simulink model "Simulatore_COMAU_UBHAND_IV_manipolazione_interna"  (Simulink 7.6)
settings: Solver=ode45  StartTime=0.0  StopTime=inf  RelTol=1e-3
values from neighbouring files (symbols this model uses):
  a_f = zeros(4,5)    [KinematicData.m]
  alpha_f = zeros(4,5)    [KinematicData.m]
  d_f = zeros(4,5)    [KinematicData.m]
  dc4_1 = 660    [KinematicData.m]
  T6HAND = [1 0 0 0;0 1 0 0; 0 0 1 357.48+95; 0 0 0 1]    [KinematicData.m]
  teta_d = atan(647.07/130)    [KinematicData.m]
  teta_f = zeros(4,5)    [KinematicData.m]
undefined symbols (not defined in the file or in its folder): a_c, a_w, alpha_c, alpha_w, d_c, d_w, Dt_fingers, teta_c
libraries: simulink x281, dspmtrx3 x171, aerolibutil x163, aerolibconvert2 x64, simulink_extras x46, vrlib x26, aerolibtransform2 x7, dspnetwork x1
(part 1 of 15)
settings Model: Creator=UMBERTO  LastModifiedBy=root
settings OptimizationCC: InitFltsAndDblsToZero=on
settings DebuggingCC: StrictBusMsg=Warning

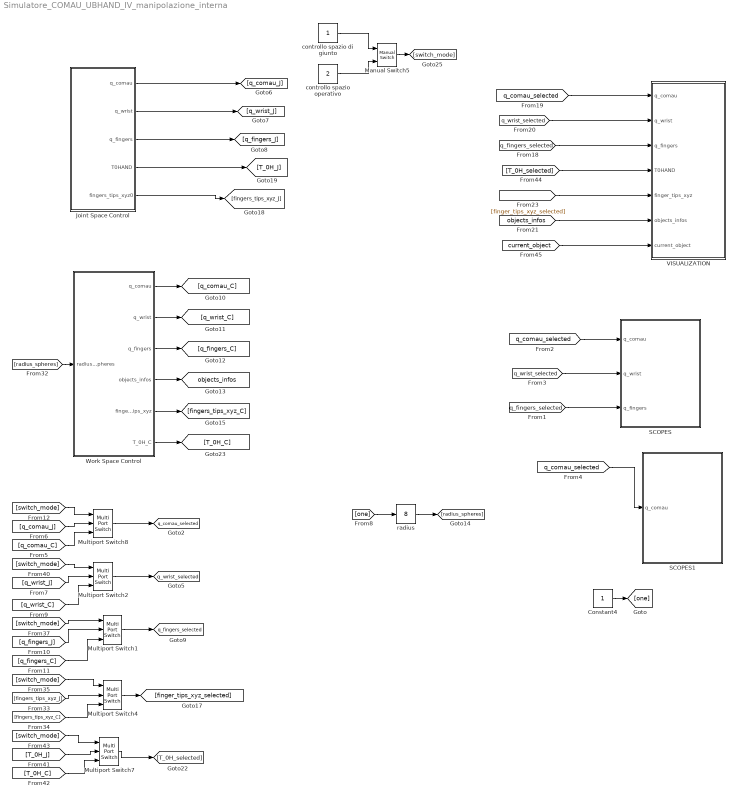
== system Simulatore_COMAU_UBHAND_IV_manipolazione_interna ==
  Constant4 : Constant
  Joint Space Control : Subsystem
  Manual Switch5 : simulink/Signal Routing/Manual Switch  SystemSampleTime=-1  sw=0  action=0  varsize=off
  Multiport Switch{1..2,4,7..8} (5 alike) : MultiPortSwitch  Inputs=2  zeroidx=off
  SCOPES : Subsystem
  SCOPES1 : Subsystem
  VISUALIZATION : Subsystem
  Work Space Control : Subsystem
  controllo spazio di giunto : Constant
  controllo spazio operativo : Constant  Value=2
  radius : simulink/Math Operations/Slider Gain  SystemSampleTime=-1  low=0  gain=8  high=200
  tag scope: objects_infos=global, q_comau_selected=global, q_fingers_selected=global, q_wrist_selected=global
  wires:
  Work Space Control/1(q_comau) -> [q_comau_C]
  Work Space Control/2(q_wrist) -> [q_wrist_C]
  Work Space Control/3(q_fingers) -> [q_fingers_C]
  Work Space Control/4(objects_infos) -> [objects_infos]
  [radius_spheres] -> Work Space Control/1(radius_spheres)
  Work Space Control/5(fingers_tips_xyz) -> [fingers_tips_xyz_C]
  Work Space Control/6(T_0H_C) -> [T_0H_C]
  Joint Space Control/1(q_comau) -> [q_comau_J]
  Joint Space Control/2(q_wrist) -> [q_wrist_J]
  Joint Space Control/3(q_fingers) -> [q_fingers_J]
  Joint Space Control/4(T0HAND) -> [T_0H_J]
  Joint Space Control/5(fingers_tips_xyz0) -> [fingers_tips_xyz_J]
  Multiport Switch8/1 -> [q_comau_selected]
  [q_comau_C] -> Multiport Switch8/3
  [q_wrist_J] -> Multiport Switch2/2
  [q_wrist_C] -> Multiport Switch2/3
  [q_fingers_J] -> Multiport Switch1/2
  [q_fingers_C] -> Multiport Switch1/3
  [switch_mode] -> Multiport Switch2/1
  [switch_mode] -> Multiport Switch1/1
  Multiport Switch1/1 -> [q_fingers_selected]
  Multiport Switch2/1 -> [q_wrist_selected]
  [fingers_tips_xyz_J] -> Multiport Switch4/2
  [fingers_tips_xyz_C] -> Multiport Switch4/3
  [switch_mode] -> Multiport Switch4/1
  Multiport Switch4/1 -> [finger_tips_xyz_selected]
  [T_0H_J] -> Multiport Switch7/2
  [T_0H_C] -> Multiport Switch7/3
  [switch_mode] -> Multiport Switch7/1
  Multiport Switch7/1 -> [T_0H_selected]
  [switch_mode] -> Multiport Switch8/1
  [q_comau_J] -> Multiport Switch8/2
  controllo spazio di giunto/1 -> Manual Switch5/1
  controllo spazio operativo/1 -> Manual Switch5/2
  Manual Switch5/1 -> [switch_mode]
  [q_comau_selected] -> VISUALIZATION/1(q_comau)
  [q_wrist_selected] -> VISUALIZATION/2(q_wrist)
  [q_fingers_selected] -> VISUALIZATION/3(q_fingers)
  [T_0H_selected] -> VISUALIZATION/4(T0HAND)
  [finger_tips_xyz_selected] -> VISUALIZATION/5(finger_tips_xyz)
  [one] -> radius/1
  radius/1 -> [radius_spheres]
  Constant4/1 -> [one]
  [q_comau_selected] -> SCOPES/1(q_comau)
  [q_wrist_selected] -> SCOPES/2(q_wrist)
  [q_fingers_selected] -> SCOPES/3(q_fingers)
  [objects_infos] -> VISUALIZATION/6(objects_infos)
  [current_object] -> VISUALIZATION/7(current_object)
  [q_comau_selected] -> SCOPES1/1(q_comau)

== system Simulatore_COMAU_UBHAND_IV_manipolazione_interna/Joint Space Control ==
  Constant : Constant
  Direct_kinematic2 : Subsystem
  Display : Display
  Mux : Mux  Inputs=6
  Mux1 : Mux  Inputs=5
  Sinusoidal Signals_fingers_wrist : Subsystem
  Step Signals fingers_wrist : Subsystem
  Switch finger references : simulink/Signal Routing/Manual Switch  sw=1  action=0  varsize=off
  Switch wrist : simulink/Signal Routing/Manual Switch  sw=1  action=0  varsize=off
  Switch{2..7} (6 alike) : simulink/Signal Routing/Manual Switch  sw=1  action=0  varsize=off
  q1 : simulink/Math Operations/Slider Gain  low=-170  gain=0  high=170
  q1_sin : Sin  Amplitude=20  Frequency=0.1  SampleTime=0
  q2 : simulink/Math Operations/Slider Gain  low=-85  gain=0  high=155
  q2_sin : Sin  Amplitude=50  Bias=20  SampleTime=0
  q3 : simulink/Math Operations/Slider Gain  low=-170  gain=-90  high=0
  q3_sin : Sin  Amplitude=35  Bias=45  Frequency=0.3  SampleTime=0
  q4 : simulink/Math Operations/Slider Gain  low=-210  gain=0  high=210
  q4_sin : Sin  Amplitude=90  Frequency=0.3  SampleTime=0
  q5 : simulink/Math Operations/Slider Gain  low=-130  gain=90  high=920
  q5_sin : Sin  Amplitude=45  Bias=45  Frequency=0.3  SampleTime=0
  q6 : simulink/Math Operations/Slider Gain  low=-2700  gain=0  high=2700
  q6_sin : Sin  Amplitude=45  Bias=45  Frequency=5  SampleTime=0
  q_comau : Outport
  q_wrist : Outport  Port=2
  q_fingers : Outport  Port=3
  T0HAND : Outport  Port=4
  fingers_tips_xyz0 : Outport  Port=5  PortDimensions=20
  wires:
  q6_sin/1 -> Switch7/2
  q5_sin/1 -> Switch6/2
  q4_sin/1 -> Switch5/2
  q3_sin/1 -> Switch4/2
  q2_sin/1 -> Switch3/2
  q1_sin/1 -> Switch2/2
  Mux/1 -> [q]
  Switch2/1 -> Mux/1
  Switch3/1 -> Mux/2
  Switch4/1 -> Mux/3
  Switch5/1 -> Mux/4
  Switch6/1 -> Mux/5
  Switch7/1 -> Mux/6
  Sinusoidal Signals_fingers_wrist/1(wrist) -> Switch wrist/2
  Switch wrist/1 -> [wrist]
  Sinusoidal Signals_fingers_wrist/2(fingers_angles) -> Switch finger references/2
  [one] -> q1/1
  Constant/1 -> [one]
  q1/1 -> Switch2/1
  [one] -> q2/1
  q2/1 -> Switch3/1
  [one] -> q3/1
  q3/1 -> Switch4/1
  [one] -> q4/1
  [one] -> q5/1
  [one] -> q6/1
  q4/1 -> Switch5/1
  q5/1 -> Switch6/1
  q6/1 -> Switch7/1
  Step Signals fingers_wrist/1(fingers_angles) -> Switch finger references/1
  Step Signals fingers_wrist/2(wrist) -> Switch wrist/1
  [q] -> q_comau/1
  [wrist] -> q_wrist/1
  [fingers] -> q_fingers/1
  Switch finger references/1 -> [fingers], Display/1
  [q] -> Direct_kinematic2/1(q[1..6])
  Direct_kinematic2/1(T0HAND) -> T0HAND/1
  [wrist] -> Direct_kinematic2/2(wrist_angles[1..2])
  [fingers] -> Direct_kinematic2/3(fingers_joints_references)
  Direct_kinematic2/2(T_finger_tip_thumb) -> Mux1/1
  Direct_kinematic2/6(T_finger_tip_little) -> Mux1/5
  Direct_kinematic2/5(T_finger_tip_ring) -> Mux1/4
  Direct_kinematic2/4(T_finger_tip_middle) -> Mux1/3
  Direct_kinematic2/3(T_finger_tip_index) -> Mux1/2
  Mux1/1 -> fingers_tips_xyz0/1

== system Simulatore_COMAU_UBHAND_IV_manipolazione_interna/SCOPES ==
  q_comau : Inport
  q_wrist : Inport  Port=2
  q_fingers : Inport  Port=3
  Demux : Demux  Outputs=5
  Demux1 : Demux  Outputs=5
  Demux2 : Demux  Outputs=6
  Direct_kinematic2 : Subsystem
  Scope : Scope  NumInputPorts=6
      settings: YMin=-70~-50~-150~-110~-90~125  YMax=-10~30~-100~-50~-20~160
  T_0HAND : Display
  T_finger_tip_index : Display
  T_finger_tip_little : Display
  T_finger_tip_middle : Display
  T_finger_tip_ring : Display
  T_finger_tip_thumb : Display
  q[1..2] wrist : Display
  q[1..4] index : Display
  q[1..4] little : Display
  q[1..4] middle : Display
  q[1..4] ring : Display
  q[1..4] thumb : Display
  q[1..6] : Display
  tag scope: T_0H=global
  wires:
  Demux/1 -> T_finger_tip_thumb/1
  Demux/2 -> T_finger_tip_index/1
  Demux/3 -> T_finger_tip_middle/1
  Demux/4 -> T_finger_tip_ring/1
  Demux/5 -> T_finger_tip_little/1
  q_comau/1 -> q[1..6]/1, Direct_kinematic2/1(q[1..6]), Demux2/1
  q_wrist/1 -> q[1..2] wrist/1, Direct_kinematic2/2(wrist_angles[1..2])
  Direct_kinematic2/2(fingers_tips_xyz) -> Demux/1
  q_fingers/1 -> Demux1/1, Direct_kinematic2/3(fingers_joints_references)
  Demux1/1 -> q[1..4] thumb/1
  Demux1/2 -> q[1..4] index/1
  Demux1/3 -> q[1..4] middle/1
  Demux1/4 -> q[1..4] ring/1
  Demux1/5 -> q[1..4] little/1
  Direct_kinematic2/1(T0HAND) -> T_0HAND/1, [T_0H]
  Demux2/1 -> Scope/1
  Demux2/2 -> Scope/2
  Demux2/3 -> Scope/3
  Demux2/6 -> Scope/6
  Demux2/4 -> Scope/4
  Demux2/5 -> Scope/5

== system Simulatore_COMAU_UBHAND_IV_manipolazione_interna/SCOPES1 ==
  q_comau : Inport
  Demux2 : Demux  Outputs=6
  Detect Change : simulink/Logic and Bit Operations/Detect Change  vinit=[6 0 0 0 0 0 0]  InputProcessing=Inherited  OutDataTypeStr=uint8
  KILL1 : Constant  Value=[6 0 0 0 0 0 0]
  Logical Operator : Logic  AllPortsSameDT=off  OutDataTypeStr=boolean
  Manual Switch1 : simulink/Signal Routing/Manual Switch  sw=0  action=0  varsize=off
  Manual Switch5 : simulink/Signal Routing/Manual Switch  sw=1  action=0  varsize=off
  Mux : Mux  Inputs=2
  NOT SEND : Constant  Value=0
  SEND : Constant
  SEND CONFIGURATION : Constant  Value=6
  Scope : Scope  NumInputPorts=6
      settings: YMin=-80~0~-175~-90~-78~80  YMax=20~100~0~20~-71~170
  Subsystem : Subsystem
  q[1..6] : Display
  wires:
  q_comau/1 -> q[1..6]/1, Demux2/1, Mux/2
  Demux2/1 -> Scope/1
  Demux2/2 -> Scope/2
  Demux2/3 -> Scope/3
  Demux2/6 -> Scope/6
  Demux2/4 -> Scope/4
  Demux2/5 -> Scope/5
  NOT SEND/1 -> Manual Switch5/1
  SEND/1 -> Manual Switch5/2
  Manual Switch5/1 -> Logical Operator/1
  Manual Switch1/1 -> Detect Change/1, Subsystem/1(DATA_TO_COMAU)
  SEND CONFIGURATION/1 -> Mux/1
  KILL1/1 -> Manual Switch1/1
  Mux/1 -> Manual Switch1/2
  Detect Change/1 -> Logical Operator/2
  Logical Operator/1 -> Subsystem/Enable

== system Simulatore_COMAU_UBHAND_IV_manipolazione_interna/VISUALIZATION ==
  q_comau : Inport
  q_wrist : Inport  Port=2
  q_fingers : Inport  Port=3
  T0HAND : Inport  Port=4
  finger_tips_xyz : Inport  Port=5
  objects_infos : Inport  Port=6
  current_object : Inport  Port=7
  Manual Switch : simulink/Signal Routing/Manual Switch  SystemSampleTime=-1  sw=0  action=0  varsize=off
  Multiport Switch1 : MultiPortSwitch  Inputs=2  zeroidx=off
  Subsystem : Subsystem
  Subsystem1 : Subsystem
  Subsystem2 : Subsystem
  Subsystem3 : Subsystem
  Subsystem4 : Subsystem
  Subsystem5 : Subsystem
  Subsystem6 : Subsystem
  Subsystem9 : Subsystem
  VR Sink1 : vrlib/VR Sink  InstantiateOnLoad=on  SampleTime=0.1  ViewEnable=on  RemoteChange=off  RemoteView=off  FieldsWritten=primo_giunto.rotation.4.1.1.double#secondo_giunto.rotation.4.1.1.double#terzo_giunto.rotation.4.1.1.double#quarto_giunto.rotation.4.1.1.double#quinto_giunto.rotation.4.1.1.double#sesto_giunto.rotation.4.1.1.double#PRIMO_GIUNTO_POLSO.rotation.4.1.1.double#SECONDO_GIUNTO_POLSO.rotation.4.1.1.double#CARPALE_POLLICE.rotation.4.1.1.double#PROSSIMALE_POLLICE.rotation.4.1.1.double#MEDIALE_POLLICE.rotation.4.1.1.double#DISTALE_POLLICE.rotation.4.1.1.double#CARPALE_INDICE.rotation.4.1.1.double#PROSSIMALE_INDICE.rotation.4.1.1.double#MEDIALE_INDICE.rotation.4.1.1.double#DISTALE_INDICE.rotation.4.1.1.double#CARPALE_MEDIO.rotation.4.1.1.double#PROSSIMALE_MEDIO.rotation.4.1.1.double#MEDIALE_MEDIO.rotation.4.1.1.double#DISTALE_MEDIO.rotation.4.1.1.double#CARPALE_ANULARE.rotation.4.1.1.double#PROSSIMALE_ANULARE.rotation.4.1.1.double#MEDIALE_ANULARE.rotation.4.1.1.double#DISTALE_ANULARE.rotation.4.1.1.double#CARPALE_MIGNOLO.rotation.4.1.1.double#PROSSIMALE_MIGNOLO.rotation.4.1.1.double#MEDIALE_MIGNOLO.rotation.4.1.1.double#DISTALE_MIGNOLO.rotation.4.1.1.double#comau_wrist.rotation.4.1.1.double#comau_wrist.translation.3.1.1.double#mignolo_punta.translation.3.1.1.double#anulare_punta.translation.3.1.1.double#medio_punta.translation.3.1.1.double#pollice_punta.translation.3.1.1.double#indice_punta.translation.3.1.1.double#system_reference_index.rotation.4.1.1.double#system_reference_index.translation.3.1.1.double#sfera.translation.3.1.1.double#sfera_size.radius.1.1.1.double#parallelepipedo.rotation.4.1.1.double#parallelepipedo.translation.3.1.1.double#parallelepipedo_size.size.3.1.1.double#cilindro.rotation.4.1.1.double#cilindro.translation.3.1.1.double#cilindro_size.height.1.1.1.double#cilindro_size.radius.1.1.1.double#mignolo_contact_point.translation.3.1.1.double#anulare_contact_point.translation.3.1.1.double#medio_contact_point.translation.3.1.1.double#pollice_contact_point.translation.3.1.1.double#indice_contact_point.translation.3.1.1.double#system_reference_1.rotation.4.1.1.double#system_reference_1.translation.3.1.1.double#system_reference_2.rotation.4.1.1.double#system_reference_2.translation.3.1.1.double#system_reference_3.rotation.4.1.1.double#system_reference_3.translation.3.1.1.double#system_reference_4.rotation.4.1.1.double#system_reference_4.translation.3.1.1.double  WorldFileName=COMAU_UBHAND_IV_cascata.WRL  WorldDescription=C:\Documents and Settings\Umberto\Documenti\Tesi\smart_six_vrml\vrml\SMART SiX_base.wrl  AutoView=on  VideoDimensions=[]  FigureProperties="{'Position', 'Name', 'Viewpoint', 'CameraBound', 'CameraDirection', 'CameraPosition', 'CameraUpVector', 'ZoomFactor', 'Antialiasing', 'Headlight', 'Lighting', 'NavPanel', 'StatusBar', 'ToolBar', 'Textures', 'Transparency', 'Wireframe', 'NavZones', 'NavMode', 'NavSpeed', 'Record2DCompressMethod', 'Record2DCompressQuality', 'Record2D', 'Record2DFileName', 'Record2DFPS'; [1.6600000000000000e+02   1.5200000000000000e+02   1.1560000000000000e+03   5.4000000000000000e+02], 'C:\\Documents and Settings\\Umberto\\Documenti\\Tesi\\smart_six_vrml\\vrml\\SMART SiX_base.wrl', 'vista_generale_da avanti', 'on', [1.3025964760090943e-02  -9.4132527063624802e-04  -9.9991471543767951e-01], [5.1898779160484864e+01   8.1516929012152815e+01  -2.5729076904413084e+01], [5.0428377425442407e-02   9.9872763980294033e-01  -2.8327345742631266e-04], [6.2092132305915493e-01], 'on', 'on', 'on', 'halfbar', 'on', 'on', 'on', 'on', 'off', 'off', 'fly', 'normal', 'auto', [7.5000000000000000e+01], 'off', '%f_anim_%n.avi', [1.5000000000000000e+01]}"
  current_object1 : InportShadow  Port=7
  wires:
  q_wrist/1 -> Subsystem3/2(q_wrist)
  q_fingers/1 -> Subsystem3/3(q_fingers)
  q_comau/1 -> Subsystem3/1(q_comau)
  T0HAND/1 -> Subsystem3/4(T_0H)
  objects_infos/1 -> Subsystem1/1(Object Infos)
  finger_tips_xyz/1 -> Subsystem3/5(finger_tips_xyz)
  current_object/1 -> Subsystem1/2(Current_object)
  Multiport Switch1/1 -> Subsystem9/1(xyz_fingers)
  [fingers_tips_xyz] -> Subsystem2/1(finger_tips_reference_into_finger_frame)
  [reference_mode] -> Multiport Switch1/1
  [T_0_index] -> Subsystem/1(T_0_index)
  current_object1/1 -> Subsystem4/1(Current_object)
  [T_0H] -> Subsystem5/1(T_0HAND)
  Subsystem2/1(finger_tips_into_base_frame) -> Manual Switch/1
  Manual Switch/1 -> Multiport Switch1/2
  Subsystem3/1(q1_comau) -> VR Sink1/1
  Subsystem3/2(q2_comau) -> VR Sink1/2
  Subsystem3/3(q3_comau) -> VR Sink1/3
  Subsystem3/4(q4_comau) -> VR Sink1/4
  Subsystem3/5(q_5_comau) -> VR Sink1/5
  Subsystem3/6(q_6_comau) -> VR Sink1/6
  Subsystem3/7(polso_1) -> VR Sink1/7
  Subsystem3/8(polso_2) -> VR Sink1/8
  Subsystem3/9(carpale_pollice) -> VR Sink1/9
  Subsystem3/10(prossimale_pollice) -> VR Sink1/10
  Subsystem3/11(mediale_pollice) -> VR Sink1/11
  Subsystem3/12(distale_pollice) -> VR Sink1/12
  Subsystem3/13(carplae_indice) -> VR Sink1/13
  Subsystem3/14(prossimale_indice) -> VR Sink1/14
  Subsystem3/15(mediale_indice) -> VR Sink1/15
  Subsystem3/16(distale_indice) -> VR Sink1/16
  Subsystem3/17(carpale_medio) -> VR Sink1/17
  Subsystem3/18(prossimale_medio) -> VR Sink1/18
  Subsystem3/19(mediale_medio) -> VR Sink1/19
  Subsystem3/20(distale_medio) -> VR Sink1/20
  Subsystem3/21(carpale_anulare) -> VR Sink1/21
  Subsystem3/22(prossimale_anulare) -> VR Sink1/22
  Subsystem3/23(mediale_anulare) -> VR Sink1/23
  Subsystem3/24(distale_anulare) -> VR Sink1/24
  Subsystem3/25(carpale_mignolo) -> VR Sink1/25
  Subsystem3/26(prossimale_mignolo) -> VR Sink1/26
  Subsystem3/27(mediale_mignolo) -> VR Sink1/27
  Subsystem3/28(distale_mignolo) -> VR Sink1/28
  Subsystem3/29(T_0H_ax/angle) -> VR Sink1/29
  Subsystem3/30(T_0H_xyz) -> VR Sink1/30
  Subsystem3/31(little_xyz) -> VR Sink1/31
  Subsystem3/32(ring_xyz) -> VR Sink1/32
  Subsystem3/33(middle_xyz) -> VR Sink1/33
  Subsystem3/34(thumb_xyz) -> VR Sink1/34
  Subsystem3/35(index_xyz) -> VR Sink1/35
  Subsystem1/1(Sphere_xyz) -> VR Sink1/38
  Subsystem1/2(sphere_radius) -> VR Sink1/39
  Subsystem1/3(Box_rotation) -> VR Sink1/40
  Subsystem1/4(Box_xyz) -> VR Sink1/41
  Subsystem1/5(Box_size) -> VR Sink1/42
  Subsystem1/6(Cylinder_rotation) -> VR Sink1/43
  Subsystem1/7(Cyliner_xyz) -> VR Sink1/44
  Subsystem1/8(Cylinder height) -> VR Sink1/45
  Subsystem1/9(Cylinder radius) -> VR Sink1/46
  Subsystem9/1(xyz_vrl_little) -> VR Sink1/47
  Subsystem9/2(xyz_vrl_ring) -> VR Sink1/48
  Subsystem9/3(xyz_vrl_middle) -> VR Sink1/49
  Subsystem9/4(xyz_vrl_thumb) -> VR Sink1/50
  Subsystem9/5(xyz_vrl_index) -> VR Sink1/51
  Subsystem4/4(T_0_rotation_frame_xyz) -> VR Sink1/57
  Subsystem4/3(T_0_rotation_frame_ax/angle) -> VR Sink1/56
  [fingers_tips_xyz_manip] -> Multiport Switch1/3
  Subsystem4/5(T_0_reference_object_frame_ax/angle) -> VR Sink1/58
  Subsystem4/6(T_0_reference_object_frame_xyz) -> VR Sink1/59
  Subsystem4/2(T_0EE_xyz) -> VR Sink1/55
  Subsystem4/1(T_0EE_ax_angle) -> VR Sink1/54
  [T_rot_center_cilindro_manip] -> Subsystem6/1(T_0HAND)
  Subsystem6/1(T_0HAND_ax/angle) -> VR Sink1/52
  Subsystem6/2(T_0HAND_xyz) -> VR Sink1/53

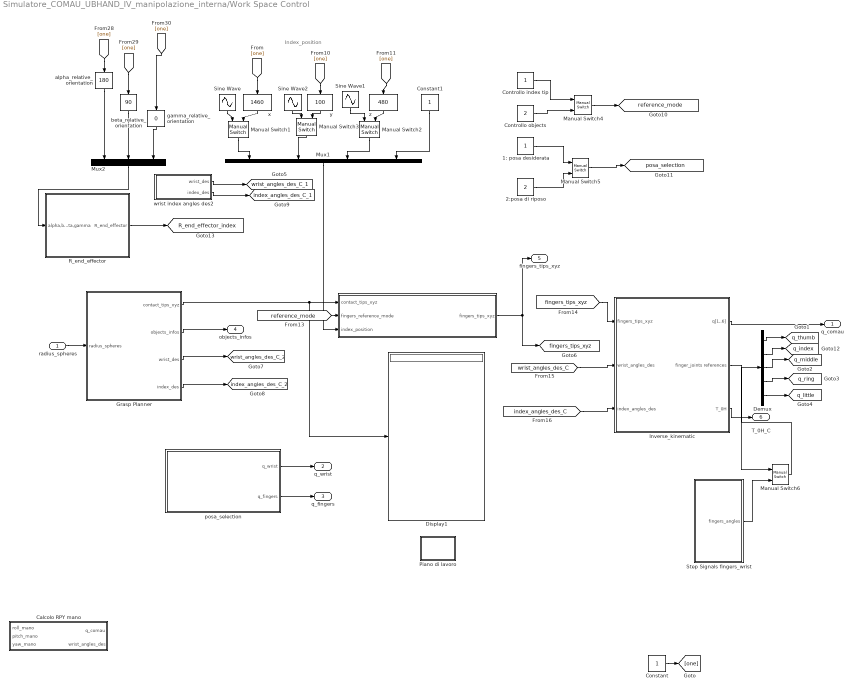
== system Simulatore_COMAU_UBHAND_IV_manipolazione_interna/Work Space Control ==
  note: Index_position
  radius_spheres : Inport
  " 2:posa di riposo" : Constant  Value=2
  " " : Subsystem
  1: posa desiderata : Constant
  Calcolo RPY mano : Subsystem
  Constant : Constant
  Constant1 : Constant
  Controllo index tip : Constant
  Controllo objects : Constant  Value=2
  Demux : Demux  Outputs=5
  Display1 : Display
  Grasp Planner : Subsystem
  Inverse_kinematic : Subsystem
  Manual Switch{1..4} (4 alike) : simulink/Signal Routing/Manual Switch  sw=0  action=0  varsize=off
  Manual Switch5 : simulink/Signal Routing/Manual Switch  sw=1  action=0  varsize=off
  Manual Switch6 : simulink/Signal Routing/Manual Switch  sw=1  action=0  varsize=off
  Mux1 : Mux
  Mux2 : Mux  Inputs=3
  Piano di lavoro : Subsystem
  R_end_effector : Subsystem
  Sine Wave : Sin  Amplitude=40  Bias=452.12  SampleTime=0
  Sine Wave1 : Sin  Amplitude=40  Bias=1200  Phase=pi/2  SampleTime=0
  Sine Wave2 : Sin  Amplitude=100  SampleTime=0
  Step Signals fingers_wrist : Subsystem
  alpha_relative_orientation : simulink/Math Operations/Slider Gain  low=-180  gain=180  high=180
  beta_relative_orientation : simulink/Math Operations/Slider Gain  low=-180  gain=90  high=180
  gamma_relative_orientation : simulink/Math Operations/Slider Gain  low=-180  gain=0  high=180
  posa_selection : Subsystem
  wrist index angles des2 : Subsystem
  x : simulink/Math Operations/Slider Gain  low=-200  gain=1460  high=2000
  y : simulink/Math Operations/Slider Gain  low=-2000  gain=100  high=2000
  z : simulink/Math Operations/Slider Gain  low=-1000  gain=480  high=2000
  q_comau : Outport
  q_wrist : Outport  Port=2
  q_fingers : Outport  Port=3
  objects_infos : Outport  Port=4
  fingers_tips_xyz : Outport  Port=5
  T_0H_C : Outport  Port=6
  tag scope: R_end_effector_index=global, fingers_tips_xyz=global, index_angles_des_C_1=global, index_angles_des_C_2=global, posa_selection=global, q_index=global, q_little=global, q_middle=global, q_ring=global, q_thumb=global, reference_mode=global, wrist_angles_des_C_1=global, wrist_angles_des_C_2=global
  wires:
  Constant/1 -> [one]
  Inverse_kinematic/1(q[1..6]) -> q_comau/1
  Grasp Planner/2(objects_infos) -> objects_infos/1
  Grasp Planner/1(contact_tips_xyz) -> " "/1(contact_tips_xyz), Display1/1
  " "/1(fingers_tips_xyz) -> fingers_tips_xyz/1, [fingers_tips_xyz]
  Demux/1 -> [q_thumb]
  radius_spheres/1 -> Grasp Planner/1(radius_spheres)
  Inverse_kinematic/3(T_0H) -> T_0H_C/1
  Grasp Planner/3(wrist_des) -> [wrist_angles_des_C_2]
  Grasp Planner/4(index_des) -> [index_angles_des_C_2]
  [one] -> z/1
  [one] -> y/1
  [one] -> x/1
  Sine Wave/1 -> Manual Switch1/1
  x/1 -> Manual Switch1/2
  Manual Switch1/1 -> Mux1/1
  Sine Wave1/1 -> Manual Switch2/1
  z/1 -> Manual Switch2/2
  Manual Switch2/1 -> Mux1/3
  Sine Wave2/1 -> Manual Switch3/1
  Manual Switch3/1 -> Mux1/2
  y/1 -> Manual Switch3/2
  Constant1/1 -> Mux1/4
  Mux1/1 -> " "/3(index_position)
  [reference_mode] -> " "/2(fingers_reference_mode)
  [fingers_tips_xyz] -> Inverse_kinematic/1("fingers_tips_xyz ")
  [wrist_angles_des_C] -> Inverse_kinematic/2(wrist_angles_des)
  [index_angles_des_C] -> Inverse_kinematic/3(index_angles_des)
  Controllo objects/1 -> Manual Switch4/2
  Controllo index tip/1 -> Manual Switch4/1
  Manual Switch4/1 -> [reference_mode]
  wrist index angles des2/1(wrist_des) -> [wrist_angles_des_C_1]
  wrist index angles des2/2(index_des) -> [index_angles_des_C_1]
  gamma_relative_orientation/1 -> Mux2/3
  beta_relative_orientation/1 -> Mux2/2
  alpha_relative_orientation/1 -> Mux2/1
  [one] -> gamma_relative_orientation/1
  [one] -> beta_relative_orientation/1
  [one] -> alpha_relative_orientation/1
  Mux2/1 -> R_end_effector/1(alpha,beta,gamma)
  R_end_effector/1(R_end_effector) -> [R_end_effector_index]
  Manual Switch5/1 -> [posa_selection]
  1: posa desiderata/1 -> Manual Switch5/1
  " 2:posa di riposo"/1 -> Manual Switch5/2
  posa_selection/1(q_wrist) -> q_wrist/1
  posa_selection/2(q_fingers) -> q_fingers/1
  Inverse_kinematic/2(finger_joints references) -> Manual Switch6/1
  Step Signals fingers_wrist/1(fingers_angles) -> Manual Switch6/2
  Manual Switch6/1 -> Demux/1
  Demux/2 -> [q_index]
  Demux/3 -> [q_middle]
  Demux/4 -> [q_ring]
  Demux/5 -> [q_little]

== system Simulatore_COMAU_UBHAND_IV_manipolazione_interna/Joint Space Control/Direct_kinematic2 ==
  q[1..6] : Inport
  wrist_angles[1..2] : Inport  Port=2
  fingers_joints_references : Inport  Port=3
  Constant2 : Constant  Value=T6HAND  VectorParams1D=off
  Direct_kinematic_Comau : Subsystem
  Direct_kinematic_hand : Subsystem
  Direct_kinematic_wrist : Subsystem
  Matrix Multiply1 : Product  Multiplication=Matrix(*)
  T0HAND : Outport
  T_finger_tip_thumb : Outport  Port=2
  T_finger_tip_index : Outport  Port=3
  T_finger_tip_middle : Outport  Port=4
  T_finger_tip_ring : Outport  Port=5
  T_finger_tip_little : Outport  Port=6
  wires:
  q[1..6]/1 -> Direct_kinematic_Comau/1(q[1..6])
  Direct_kinematic_Comau/1(T_0_6) -> Matrix Multiply1/1
  Constant2/1 -> Matrix Multiply1/2
  Matrix Multiply1/1 -> Direct_kinematic_wrist/2(T_0HAND), T0HAND/1
  wrist_angles[1..2]/1 -> Direct_kinematic_wrist/1(wrist_angles[1..2])
  fingers_joints_references/1 -> Direct_kinematic_hand/1(Joint_references)
  Direct_kinematic_hand/1(T_finger_tip_thumb) -> T_finger_tip_thumb/1
  Direct_kinematic_hand/2(T_finger_tip_index) -> T_finger_tip_index/1
  Direct_kinematic_hand/3(T_finger_tip_middle) -> T_finger_tip_middle/1
  Direct_kinematic_hand/4(T_finger_tip_ring) -> T_finger_tip_ring/1
  Direct_kinematic_hand/5(T_finger_tip_little) -> T_finger_tip_little/1
  Direct_kinematic_wrist/1(T_08) -> Direct_kinematic_hand/2(T_08)

== system Simulatore_COMAU_UBHAND_IV_manipolazione_interna/Joint Space Control/Sinusoidal Signals_fingers_wrist ==
  Index_medial : Sin  Amplitude=45  Bias=45  Phase=3/2*pi  SampleTime=0
  Index_proximal_back_forward : Sin  Amplitude=45  Bias=45  Phase=3/2*pi  SampleTime=0
  Index_proximal_left_right : Constant  Value=0
  Little_medial : Sin  Amplitude=0  Phase=3/2*pi  SampleTime=0
  Little_proximal_back_forward : Sin  Amplitude=0  Phase=3/2*pi  SampleTime=0
  Little_proximal_left_right : Constant  Value=0
  Middle_medial : Sin  Amplitude=0  Phase=3/2*pi  SampleTime=0
  Middle_proximal_back_forward : Sin  Amplitude=0  Phase=3/2*pi  SampleTime=0
  Middle_proximal_left_right : Constant  Value=0
  Mux{1..5,7} (6 alike) : Mux  Inputs=3
  Mux6 : Mux  Inputs=2
  Mux8 : Mux  Inputs=5
  Mux9 : Mux  Inputs=5
  Ring_medial : Sin  Amplitude=0  Phase=3/2*pi  SampleTime=0
  Ring_proximal_back_forward : Sin  Amplitude=0  Phase=3/2*pi  SampleTime=0
  Ring_proximal_left_right : Constant  Value=0
  Switch wrist : simulink/Signal Routing/Manual Switch  sw=0  action=0  varsize=off
  Thumb_medial : Sin  Amplitude=0  Phase=3/2*pi  SampleTime=0
  Thumb_medial1 : Sin  Amplitude=0  Phase=3/2*pi  SampleTime=0
  Thumb_proximal_back_forward : Sin  Amplitude=35  Bias=35  Phase=3/2*pi  SampleTime=0
  Thumb_proximal_back_forward1 : Sin  Amplitude=45  Bias=45  Phase=3/2*pi  SampleTime=0
  Thumb_proximal_left_right : Constant  Value=0
  Thumb_proximal_left_right1 : Sin  Amplitude=20  Phase=3/2*pi  SampleTime=0
  Wrist_back_forward : Sin  Amplitude=30  SampleTime=0
  Wrist_left_right : Sin  Amplitude=30  Phase=pi/2  SampleTime=0
  wrist : Outport
  fingers_angles : Outport  Port=2
  wires:
  Thumb_proximal_left_right/1 -> Mux1/1
  Thumb_medial/1 -> Mux1/3
  Mux6/1 -> wrist/1
  Mux1/1 -> [thumb], Mux9/1
  Thumb_proximal_back_forward/1 -> Mux1/2
  Index_proximal_left_right/1 -> Mux2/1
  Index_medial/1 -> Mux2/3
  Mux2/1 -> [index]
  Index_proximal_back_forward/1 -> Mux2/2
  Middle_proximal_left_right/1 -> Mux3/1
  Middle_medial/1 -> Mux3/3
  Mux3/1 -> [middle]
  Middle_proximal_back_forward/1 -> Mux3/2
  Ring_proximal_left_right/1 -> Mux4/1
  Ring_medial/1 -> Mux4/3
  Mux4/1 -> [ring]
  Ring_proximal_back_forward/1 -> Mux4/2
  Little_proximal_left_right/1 -> Mux5/1
  Little_medial/1 -> Mux5/3
  Mux5/1 -> [little]
  Little_proximal_back_forward/1 -> Mux5/2
  Wrist_left_right/1 -> Mux6/1
  Wrist_back_forward/1 -> Mux6/2
  Mux7/1 -> Mux9/3, Mux9/2, Mux9/4, Mux9/5
  Thumb_medial1/1 -> Mux7/3
  Thumb_proximal_back_forward1/1 -> Mux7/2
  Mux9/1 -> Switch wrist/2
  Mux8/1 -> Switch wrist/1
  Switch wrist/1 -> fingers_angles/1
  [thumb] -> Mux8/1
  [index] -> Mux8/2
  [middle] -> Mux8/3
  [ring] -> Mux8/4
  [little] -> Mux8/5
  Thumb_proximal_left_right1/1 -> Mux7/1

== system Simulatore_COMAU_UBHAND_IV_manipolazione_interna/Joint Space Control/Step Signals fingers_wrist ==
  All_fingers_together_back_forward : Constant  Value=0
  All_fingers_together_medial : Constant  Value=0
  All_fingers_together_proximal_left_right : Constant  Value=0
  Index_medial : Constant  Value=40
  Index_proximal_back_forward : Constant  Value=30
  Index_proximal_left_right : Constant  Value=5
  Little_medial : Constant  Value=0
  Little_proximal_back_forward : Constant  Value=0
  Little_proximal_left_right : Constant  Value=0
  Middle_medial : Constant  Value=30
  Middle_proximal_back_forward : Constant  Value=45
  Middle_proximal_left_right : Constant  Value=0
  Mux{1..5,7} (6 alike) : Mux  Inputs=3
  Mux6 : Mux  Inputs=2
  Mux8 : Mux  Inputs=5
  Mux9 : Mux  Inputs=5
  Ring_medial : Constant  Value=0
  Ring_proximal_back_forward : Constant  Value=0
  Ring_proximal_left_right : Constant  Value=0
  Switch wrist : simulink/Signal Routing/Manual Switch  sw=0  action=0  varsize=off
  Thumb_medial : Constant  Value=0
  Thumb_proximal_back_forward : Constant  Value=50
  Thumb_proximal_left_right : Constant  Value=0
  Wrist_back_forward1 : Constant  Value=0
  Wrist_left_right : Constant  Value=0
  fingers_angles : Outport
  wrist : Outport  Port=2
  tag scope: index_angles_des_J=global
  wires:
  Mux6/1 -> wrist/1
  Index_proximal_left_right/1 -> Mux2/1
  Index_medial/1 -> Mux2/3
  Index_proximal_back_forward/1 -> Mux2/2
  Middle_proximal_left_right/1 -> Mux3/1
  Middle_medial/1 -> Mux3/3
  Middle_proximal_back_forward/1 -> Mux3/2
  Ring_proximal_left_right/1 -> Mux4/1
  Ring_medial/1 -> Mux4/3
  Ring_proximal_back_forward/1 -> Mux4/2
  Little_proximal_left_right/1 -> Mux5/1
  Little_medial/1 -> Mux5/3
  Little_proximal_back_forward/1 -> Mux5/2
  Mux1/1 -> [thumb], Mux9/1
  Thumb_proximal_left_right/1 -> Mux1/1
  Thumb_medial/1 -> Mux1/3
  Thumb_proximal_back_forward/1 -> Mux1/2
  Mux2/1 -> [index], [index_angles_des_J]
  Mux3/1 -> [middle]
  Mux4/1 -> [ring]
  Mux5/1 -> [little]
  Wrist_left_right/1 -> Mux6/1
  Wrist_back_forward1/1 -> Mux6/2
  Mux7/1 -> Mux9/3, Mux9/2, Mux9/5, Mux9/4
  All_fingers_together_proximal_left_right/1 -> Mux7/1
  All_fingers_together_medial/1 -> Mux7/3
  All_fingers_together_back_forward/1 -> Mux7/2
  Mux9/1 -> Switch wrist/2
  Mux8/1 -> Switch wrist/1
  Switch wrist/1 -> fingers_angles/1
  [thumb] -> Mux8/1
  [index] -> Mux8/2
  [middle] -> Mux8/3
  [ring] -> Mux8/4
  [little] -> Mux8/5

== system Simulatore_COMAU_UBHAND_IV_manipolazione_interna/SCOPES/Direct_kinematic2 ==
  q[1..6] : Inport
  wrist_angles[1..2] : Inport  Port=2
  fingers_joints_references : Inport  Port=3
  Constant2 : Constant  Value=T6HAND  VectorParams1D=off
  Direct_kinematic_Comau : Subsystem
  Direct_kinematic_hand : Subsystem
  Direct_kinematic_wrist : Subsystem
  Matrix Multiply1 : Product  Multiplication=Matrix(*)
  Mux : Mux  Inputs=5
  T0HAND : Outport
  fingers_tips_xyz : Outport  Port=2
  tag scope: T_PALMO=global
  wires:
  q[1..6]/1 -> Direct_kinematic_Comau/1(q[1..6])
  Direct_kinematic_Comau/1(T_0_6) -> Matrix Multiply1/1
  Constant2/1 -> Matrix Multiply1/2
  Matrix Multiply1/1 -> Direct_kinematic_wrist/2(T_0HAND), T0HAND/1
  wrist_angles[1..2]/1 -> Direct_kinematic_wrist/1(wrist_angles[1..2])
  fingers_joints_references/1 -> Direct_kinematic_hand/1(Joint_references)
  Direct_kinematic_wrist/1(T_08) -> Direct_kinematic_hand/2(T_08), [T_PALMO]
  Direct_kinematic_hand/1(T_finger_tip_thumb) -> Mux/1
  Direct_kinematic_hand/2(T_finger_tip_index) -> Mux/2
  Direct_kinematic_hand/3(T_finger_tip_middle) -> Mux/3
  Direct_kinematic_hand/4(T_finger_tip_ring) -> Mux/4
  Direct_kinematic_hand/5(T_finger_tip_little) -> Mux/5
  Mux/1 -> fingers_tips_xyz/1

== system Simulatore_COMAU_UBHAND_IV_manipolazione_interna/SCOPES1/Subsystem ==
  DATA_TO_COMAU : Inport
  Enable : EnablePort
  UDP Send : dspnetwork/UDP Send  remoteURL='192.168.7.30'  remotePort=3456  localURL='255.255.255.255'  localPortSource=Automatically determine  localPort=-1  sampletime=-1  blockPlatform=LINUX  separateLengthPort=0
  wires:
  DATA_TO_COMAU/1 -> UDP Send/1

== system Simulatore_COMAU_UBHAND_IV_manipolazione_interna/VISUALIZATION/Subsystem ==
  T_0_index : Inport  PortDimensions=[4 4]
  Demux15 : Demux
  Demux16 : Demux
  Gain6 : Gain  Gain=-1
  Gain7 : Gain  Gain=-1
  Mux6 : Mux
  Mux7 : Mux
  Rotation Matrix to VRML Rotation3 : vrlib/Utilities/Rotation Matrix to VRML Rotation  maxzero=1e-12
  Submatrix3 : dspmtrx3/Submatrix  RowSpan=Range of rows  RowStartMode=Index  RowStartIndex=1  RowEndMode=Index  RowEndIndex=3  ColSpan=Range of columns  ColStartMode=Index  ColStartIndex=1  ColEndMode=Index  ColEndIndex=3
  Submatrix4 : dspmtrx3/Submatrix  RowSpan=Range of rows  RowStartMode=Index  RowStartIndex=1  RowEndMode=Index  RowEndIndex=3  ColSpan=One column  ColStartMode=Index  ColStartIndex=4  ColEndMode=Index  ColEndIndex=3
  sphere_xyz1 : Subsystem
  T_0_index_ax/angle : Outport
  T_0_index_xyz : Outport  Port=2
  wires:
  Rotation Matrix to VRML Rotation3/1 -> Demux16/1
  Demux16/4 -> Mux6/4
  Demux16/3 -> Mux6/2
  Mux6/1 -> Demux15/1
  Demux15/1 -> Mux7/1
  Demux15/2 -> Mux7/2
  Demux15/3 -> Mux7/3
  Demux15/4 -> Mux7/4
  Demux16/1 -> Gain6/1
  Demux16/2 -> Gain7/1
  Gain6/1 -> Mux6/3
  Gain7/1 -> Mux6/1
  Submatrix3/1 -> Rotation Matrix to VRML Rotation3/1
  Submatrix4/1 -> sphere_xyz1/1(xyz_0)
  Mux7/1 -> T_0_index_ax/angle/1
  sphere_xyz1/1(xyz_vrl) -> T_0_index_xyz/1
  T_0_index/1 -> Submatrix3/1, Submatrix4/1

== system Simulatore_COMAU_UBHAND_IV_manipolazione_interna/VISUALIZATION/Subsystem1 ==
  Object Infos : Inport
  Current_object : Inport  Port=2
  Bus Selector : BusSelector  OutputSignals=signal1,signal2,signal3,signal4,signal5,signal6
  Demux{9..14} (6 alike) : Demux
  Demux8 : Demux  Outputs=2
  Gain{,1..5} (6 alike) : Gain  Gain=-1
  Multiport Switch1 : MultiPortSwitch  zeroidx=off
  Mux{,1..5} (6 alike) : Mux
  Rotation Matrix to VRML Rotation : vrlib/Utilities/Rotation Matrix to VRML Rotation  maxzero=1e-12
  Rotation Matrix to VRML Rotation1 : vrlib/Utilities/Rotation Matrix to VRML Rotation  maxzero=1e-12
  Rotation Matrix to VRML Rotation2 : vrlib/Utilities/Rotation Matrix to VRML Rotation  maxzero=1e-12
  box_xyz : Subsystem
  cilindro_xyz : Subsystem
  sphere_xyz : Subsystem
  Sphere_xyz : Outport
  sphere_radius : Outport  Port=2
  Box_rotation : Outport  Port=3
  Box_xyz : Outport  Port=4
  Box_size : Outport  Port=5
  Cylinder_rotation : Outport  Port=6
  Cyliner_xyz : Outport  Port=7
  Cylinder height : Outport  Port=8
  Cylinder radius : Outport  Port=9
  System_ref_rotation : Outport  Port=10
  wires:
  Bus Selector/1 -> sphere_xyz/1(xyz_0)  "<signal1>"
  Bus Selector/3 -> box_xyz/1(xyz_0)  "<signal3>"
  Bus Selector/5 -> cilindro_xyz/1(xyz_0)  "<signal5>"
  Bus Selector/6 -> Demux8/1  "<signal6>"
  sphere_xyz/1(xyz_vrl) -> Sphere_xyz/1
  Bus Selector/2 -> sphere_radius/1  "<signal2>"
  Object Infos/1 -> Bus Selector/1
  Bus Selector/4 -> Box_size/1  "<signal4>"
  box_xyz/1(xyz_vrl) -> Box_xyz/1
  cilindro_xyz/1(xyz_vrl) -> Cyliner_xyz/1
  Demux8/1 -> Cylinder height/1
  Demux8/2 -> Cylinder radius/1
  Rotation Matrix to VRML Rotation/1 -> Demux9/1
  Demux9/4 -> Mux/4
  Demux9/3 -> Mux/2
  Mux/1 -> Demux10/1
  Demux10/1 -> Mux1/1
  Demux10/2 -> Mux1/2
  Demux10/3 -> Mux1/3
  Demux10/4 -> Mux1/4
  Demux9/1 -> Gain/1
  Demux9/2 -> Gain1/1
  Gain/1 -> Mux/3
  Gain1/1 -> Mux/1
  [R_sfera] -> Rotation Matrix to VRML Rotation/1
  Gain3/1 -> Mux2/1
  Gain2/1 -> Mux2/3
  Demux12/2 -> Gain3/1
  Demux11/4 -> Mux3/4
  Demux11/3 -> Mux3/3
  Demux11/2 -> Mux3/2
  Mux2/1 -> Demux11/1
  Demux12/3 -> Mux2/2
  Demux12/4 -> Mux2/4
  Rotation Matrix to VRML Rotation1/1 -> Demux12/1
  Demux12/1 -> Gain2/1
  Demux11/1 -> Mux3/1
  Mux1/1 -> Multiport Switch1/2
  Mux3/1 -> Multiport Switch1/4, Cylinder_rotation/1
  [R_box] -> Rotation Matrix to VRML Rotation2/1
  Gain5/1 -> Mux4/1
  Gain4/1 -> Mux4/3
  Demux14/2 -> Gain5/1
  Demux13/4 -> Mux5/4
  Demux13/3 -> Mux5/3
  Demux13/2 -> Mux5/2
  Mux4/1 -> Demux13/1
  Demux14/3 -> Mux4/2
  Demux14/4 -> Mux4/4
  Rotation Matrix to VRML Rotation2/1 -> Demux14/1
  Demux14/1 -> Gain4/1
  Demux13/1 -> Mux5/1
  Mux5/1 -> Multiport Switch1/3, Box_rotation/1
  Current_object/1 -> Multiport Switch1/1
  Multiport Switch1/1 -> System_ref_rotation/1
  [R_cilindro] -> Rotation Matrix to VRML Rotation1/1

== system Simulatore_COMAU_UBHAND_IV_manipolazione_interna/VISUALIZATION/Subsystem2 ==
  finger_tips_reference_into_finger_frame : Inport
  Demux : Demux  Outputs=20
  Matrix Multiply{,1..4} (5 alike) : Product  Multiplication=Matrix(*)
  Mux1 : Mux  Inputs=5
  Mux{2..4,6..7} (5 alike) : Mux
  finger_tips_into_base_frame : Outport
  wires:
  Demux/1 -> Mux3/1
  Demux/2 -> Mux3/2
  Demux/3 -> Mux3/3
  Demux/4 -> Mux3/4
  Demux/5 -> Mux4/1
  Demux/6 -> Mux4/2
  Demux/7 -> Mux4/3
  Demux/8 -> Mux4/4
  Demux/9 -> Mux6/1
  Demux/10 -> Mux6/2
  Demux/11 -> Mux6/3
  Demux/12 -> Mux6/4
  Demux/13 -> Mux7/1
  Demux/14 -> Mux7/2
  Demux/15 -> Mux7/3
  Demux/16 -> Mux7/4
  [T_0_thumb] -> Matrix Multiply/1
  Mux3/1 -> Matrix Multiply/2
  [T_0_middle] -> Matrix Multiply2/1
  [T_0_ring] -> Matrix Multiply3/1
  [T_0_little] -> Matrix Multiply4/1
  Matrix Multiply/1 -> Mux1/1
  Mux1/1 -> finger_tips_into_base_frame/1
  finger_tips_reference_into_finger_frame/1 -> Demux/1
  Demux/17 -> Mux2/1
  Demux/18 -> Mux2/2
  Demux/19 -> Mux2/3
  Demux/20 -> Mux2/4
  Mux2/1 -> Matrix Multiply4/2
  Mux7/1 -> Matrix Multiply3/2
  Mux6/1 -> Matrix Multiply2/2
  Mux4/1 -> Matrix Multiply1/2
  [T_0_index] -> Matrix Multiply1/1
  Matrix Multiply1/1 -> Mux1/2
  Matrix Multiply4/1 -> Mux1/5
  Matrix Multiply2/1 -> Mux1/3
  Matrix Multiply3/1 -> Mux1/4

== system Simulatore_COMAU_UBHAND_IV_manipolazione_interna/VISUALIZATION/Subsystem3 ==
  q_comau : Inport
  q_wrist : Inport  Port=2
  q_fingers : Inport  Port=3
  T_0H : Inport  Port=4
  finger_tips_xyz : Inport  Port=5
  Demux : Demux  Outputs=5
  Subsystem1 : Subsystem
  Subsystem2 : Subsystem
  finger_tip_xyz : Subsystem
  q1_comau : Outport
  q2_comau : Outport  Port=2
  q3_comau : Outport  Port=3
  q4_comau : Outport  Port=4
  q_5_comau : Outport  Port=5
  q_6_comau : Outport  Port=6
  polso_1 : Outport  Port=7
  polso_2 : Outport  Port=8
  carpale_pollice : Outport  Port=9
  prossimale_pollice : Outport  Port=10
  mediale_pollice : Outport  Port=11
  distale_pollice : Outport  Port=12
  carplae_indice : Outport  Port=13
  prossimale_indice : Outport  Port=14
  mediale_indice : Outport  Port=15
  distale_indice : Outport  Port=16
  carpale_medio : Outport  Port=17
  prossimale_medio : Outport  Port=18
  mediale_medio : Outport  Port=19
  distale_medio : Outport  Port=20
  carpale_anulare : Outport  Port=21
  prossimale_anulare : Outport  Port=22
  mediale_anulare : Outport  Port=23
  distale_anulare : Outport  Port=24
  carpale_mignolo : Outport  Port=25
  prossimale_mignolo : Outport  Port=26
  mediale_mignolo : Outport  Port=27
  distale_mignolo : Outport  Port=28
  T_0H_ax/angle : Outport  Port=29
  T_0H_xyz : Outport  Port=30
  little_xyz : Outport  Port=31
  ring_xyz : Outport  Port=32
  middle_xyz : Outport  Port=33
  thumb_xyz : Outport  Port=34
  index_xyz : Outport  Port=35
  wires:
  Subsystem2/1(q1_out) -> q1_comau/1
  Subsystem2/2(q2_out) -> q2_comau/1
  Subsystem2/3(q3_out) -> q3_comau/1
  Subsystem2/4(q4_out) -> q4_comau/1
  q_comau/1 -> Subsystem2/1(q_comau)
  Subsystem2/5(q5_out) -> q_5_comau/1
  Subsystem2/6(q6_out) -> q_6_comau/1
  Subsystem2/7(polso_1) -> polso_1/1
  Subsystem2/8(polso_2) -> polso_2/1
  Subsystem2/9(carpale_pollice) -> carpale_pollice/1
  Subsystem2/10(prossimale_pollice) -> prossimale_pollice/1
  Subsystem2/11(mediale_pollice) -> mediale_pollice/1
  Subsystem2/12(distale_pollice) -> distale_pollice/1
  Subsystem2/13(carpale_indice) -> carplae_indice/1
  Subsystem2/14(prossimale_indice) -> prossimale_indice/1
  q_wrist/1 -> Subsystem2/2(q_wrist)
  Subsystem2/15(mediale_indice) -> mediale_indice/1
  Subsystem2/16(distale_indice) -> distale_indice/1
  Subsystem2/17(carpale_medio) -> carpale_medio/1
  Subsystem2/18(prossimale_medio) -> prossimale_medio/1
  Subsystem2/19(mediale_medio) -> mediale_medio/1
  Subsystem2/20(distale_medio) -> distale_medio/1
  Subsystem2/21(carpale_anulare) -> carpale_anulare/1
  Subsystem2/22(prossimale_anulare) -> prossimale_anulare/1
  Subsystem2/23(mediale_anulare) -> mediale_anulare/1
  Subsystem2/24(distale_anulare) -> distale_anulare/1
  q_fingers/1 -> Subsystem2/3(q_fingers)
  Subsystem2/25(carpale_mignolo) -> carpale_mignolo/1
  Subsystem2/26(prossimale_mignolo) -> prossimale_mignolo/1
  Subsystem2/27(mediale_mignolo) -> mediale_mignolo/1
  Subsystem2/28(distale_mignolo) -> distale_mignolo/1
  Subsystem1/1(ax/angle_vrl_wrist) -> T_0H_ax/angle/1
  T_0H/1 -> Subsystem1/1(TOHAND)
  Subsystem1/2(xyz_vrl_wrist) -> T_0H_xyz/1
  finger_tip_xyz/1(vrl_xyz_thumb) -> thumb_xyz/1
  finger_tip_xyz/2(vrl_xyz_index) -> index_xyz/1
  finger_tip_xyz/3(vrl_xyz_middle) -> middle_xyz/1
  finger_tip_xyz/4(vrl_xyz_ring) -> ring_xyz/1
  finger_tip_xyz/5(vrl_xyz_little) -> little_xyz/1
  finger_tips_xyz/1 -> Demux/1
  Demux/1 -> finger_tip_xyz/1(T_finger_tip_thumb)
  Demux/2 -> finger_tip_xyz/2(T_finger_tip_index)
  Demux/3 -> finger_tip_xyz/3(T_finger_tip_middle)
  Demux/4 -> finger_tip_xyz/4(T_finger_tip_ring)
  Demux/5 -> finger_tip_xyz/5(T_finger_tip_little)

== system Simulatore_COMAU_UBHAND_IV_manipolazione_interna/VISUALIZATION/Subsystem4 ==
  Current_object : Inport
  Current_object1 : InportShadow  Port=1
  Current_object2 : InportShadow  Port=1
  Demux{15..16,21..24} (6 alike) : Demux
  Gain{6..7,12..15} (6 alike) : Gain  Gain=-1
  Multiport Switch3 : MultiPortSwitch  zeroidx=off
  Multiport Switch4 : MultiPortSwitch  zeroidx=off
  Multiport Switch5 : MultiPortSwitch  zeroidx=off
  Mux{6..7,12..15} (6 alike) : Mux
  Rotation Matrix to VRML Rotation3 : vrlib/Utilities/Rotation Matrix to VRML Rotation  maxzero=1e-12
  Rotation Matrix to VRML Rotation6 : vrlib/Utilities/Rotation Matrix to VRML Rotation  maxzero=1e-12
  Rotation Matrix to VRML Rotation7 : vrlib/Utilities/Rotation Matrix to VRML Rotation  maxzero=1e-12
  Submatrix3 : dspmtrx3/Submatrix  RowSpan=Range of rows  RowStartMode=Index  RowStartIndex=1  RowEndMode=Index  RowEndIndex=3  ColSpan=Range of columns  ColStartMode=Index  ColStartIndex=1  ColEndMode=Index  ColEndIndex=3
  Submatrix4 : dspmtrx3/Submatrix  RowSpan=Range of rows  RowStartMode=Index  RowStartIndex=1  RowEndMode=Index  RowEndIndex=3  ColSpan=One column  ColStartMode=Index  ColStartIndex=4  ColEndMode=Index  ColEndIndex=3
  Submatrix5 : dspmtrx3/Submatrix  RowSpan=Range of rows  RowStartMode=Index  RowStartIndex=1  RowEndMode=Index  RowEndIndex=3  ColSpan=Range of columns  ColStartMode=Index  ColStartIndex=1  ColEndMode=Index  ColEndIndex=3
  Submatrix6 : dspmtrx3/Submatrix  RowSpan=Range of rows  RowStartMode=Index  RowStartIndex=1  RowEndMode=Index  RowEndIndex=3  ColSpan=One column  ColStartMode=Index  ColStartIndex=4  ColEndMode=Index  ColEndIndex=3
  sphere_xyz1 : Subsystem
  sphere_xyz3 : Subsystem
  sphere_xyz4 : Subsystem
  T_0EE_ax_angle : Outport
  T_0EE_xyz : Outport  Port=2
  T_0_rotation_frame_ax/angle : Outport  Port=3
  T_0_rotation_frame_xyz : Outport  Port=4
  T_0_reference_object_frame_ax/angle : Outport  Port=5
  T_0_reference_object_frame_xyz : Outport  Port=6
  wires:
  Rotation Matrix to VRML Rotation3/1 -> Demux16/1
  Demux16/4 -> Mux6/4
  Demux16/3 -> Mux6/2
  Mux6/1 -> Demux15/1
  Demux15/1 -> Mux7/1
  Demux15/2 -> Mux7/2
  Demux15/3 -> Mux7/3
  Demux15/4 -> Mux7/4
  Demux16/1 -> Gain6/1
  Demux16/2 -> Gain7/1
  Gain6/1 -> Mux6/3
  Gain7/1 -> Mux6/1
  Submatrix3/1 -> Rotation Matrix to VRML Rotation3/1
  Mux7/1 -> T_0EE_ax_angle/1
  Submatrix4/1 -> sphere_xyz1/1(xyz_0)
  [T_0EE] -> Submatrix3/1, Submatrix4/1
  Rotation Matrix to VRML Rotation6/1 -> Demux22/1
  Demux22/4 -> Mux12/4
  Demux22/3 -> Mux12/2
  Mux12/1 -> Demux21/1
  Demux21/1 -> Mux13/1
  Demux21/2 -> Mux13/2
  Demux21/3 -> Mux13/3
  Demux21/4 -> Mux13/4
  Demux22/1 -> Gain12/1
  Demux22/2 -> Gain13/1
  Gain12/1 -> Mux12/3
  Gain13/1 -> Mux12/1
  Submatrix5/1 -> Rotation Matrix to VRML Rotation6/1
  Mux13/1 -> T_0_rotation_frame_ax/angle/1
  Submatrix6/1 -> sphere_xyz3/1(xyz_0)
  sphere_xyz3/1(xyz_vrl) -> T_0_rotation_frame_xyz/1
  Rotation Matrix to VRML Rotation7/1 -> Demux24/1
  Demux24/4 -> Mux14/4
  Demux24/3 -> Mux14/2
  Mux14/1 -> Demux23/1
  Demux23/1 -> Mux15/1
  Demux23/2 -> Mux15/2
  Demux23/3 -> Mux15/3
  Demux23/4 -> Mux15/4
  Demux24/1 -> Gain14/1
  Demux24/2 -> Gain15/1
  Gain14/1 -> Mux14/3
  Gain15/1 -> Mux14/1
  Mux15/1 -> T_0_reference_object_frame_ax/angle/1
  sphere_xyz4/1(xyz_vrl) -> T_0_reference_object_frame_xyz/1
  [T_rot_center_sfera] -> Multiport Switch3/2
  [T_rot_center_box] -> Multiport Switch3/3
  [T_rot_center_cilindro] -> Multiport Switch3/4
  Multiport Switch3/1 -> Submatrix6/1, Submatrix5/1
  [R_sfera] -> Multiport Switch4/2
  [R_box] -> Multiport Switch4/3
  Multiport Switch4/1 -> Rotation Matrix to VRML Rotation7/1
  [reference_point_cilindro] -> Multiport Switch5/4
  [reference_point_box] -> Multiport Switch5/3
  [reference_point_sfera] -> Multiport Switch5/2
  Multiport Switch5/1 -> sphere_xyz4/1(xyz_0)
  sphere_xyz1/1(xyz_vrl) -> T_0EE_xyz/1
  Current_object/1 -> Multiport Switch3/1
  Current_object2/1 -> Multiport Switch5/1
  Current_object1/1 -> Multiport Switch4/1
  [R_cilindro] -> Multiport Switch4/4

== system Simulatore_COMAU_UBHAND_IV_manipolazione_interna/VISUALIZATION/Subsystem5 ==
  T_0HAND : Inport  PortDimensions=[4 4]
  Demux15 : Demux
  Demux16 : Demux
  Gain6 : Gain  Gain=-1
  Gain7 : Gain  Gain=-1
  Mux6 : Mux
  Mux7 : Mux
  Rotation Matrix to VRML Rotation3 : vrlib/Utilities/Rotation Matrix to VRML Rotation  maxzero=1e-12
  Submatrix3 : dspmtrx3/Submatrix  RowSpan=Range of rows  RowStartMode=Index  RowStartIndex=1  RowEndMode=Index  RowEndIndex=3  ColSpan=Range of columns  ColStartMode=Index  ColStartIndex=1  ColEndMode=Index  ColEndIndex=3
  Submatrix4 : dspmtrx3/Submatrix  RowSpan=Range of rows  RowStartMode=Index  RowStartIndex=1  RowEndMode=Index  RowEndIndex=3  ColSpan=One column  ColStartMode=Index  ColStartIndex=4  ColEndMode=Index  ColEndIndex=3
  sphere_xyz1 : Subsystem
  T_0HAND_ax/angle : Outport
  T_0HAND_xyz : Outport  Port=2
  wires:
  T_0HAND/1 -> Submatrix4/1, Submatrix3/1
  sphere_xyz1/1(xyz_vrl) -> T_0HAND_xyz/1
  Mux7/1 -> T_0HAND_ax/angle/1
  Submatrix4/1 -> sphere_xyz1/1(xyz_0)
  Submatrix3/1 -> Rotation Matrix to VRML Rotation3/1
  Gain7/1 -> Mux6/1
  Gain6/1 -> Mux6/3
  Demux16/2 -> Gain7/1
  Demux16/1 -> Gain6/1
  Demux15/4 -> Mux7/4
  Demux15/3 -> Mux7/3
  Demux15/2 -> Mux7/2
  Demux15/1 -> Mux7/1
  Mux6/1 -> Demux15/1
  Demux16/3 -> Mux6/2
  Demux16/4 -> Mux6/4
  Rotation Matrix to VRML Rotation3/1 -> Demux16/1

== system Simulatore_COMAU_UBHAND_IV_manipolazione_interna/VISUALIZATION/Subsystem6 ==
  T_0HAND : Inport  PortDimensions=[4 4]
  Demux15 : Demux
  Demux16 : Demux
  Gain6 : Gain  Gain=-1
  Gain7 : Gain  Gain=-1
  Mux6 : Mux
  Mux7 : Mux
  Rotation Matrix to VRML Rotation3 : vrlib/Utilities/Rotation Matrix to VRML Rotation  maxzero=1e-12
  Submatrix3 : dspmtrx3/Submatrix  RowSpan=Range of rows  RowStartMode=Index  RowStartIndex=1  RowEndMode=Index  RowEndIndex=3  ColSpan=Range of columns  ColStartMode=Index  ColStartIndex=1  ColEndMode=Index  ColEndIndex=3
  Submatrix4 : dspmtrx3/Submatrix  RowSpan=Range of rows  RowStartMode=Index  RowStartIndex=1  RowEndMode=Index  RowEndIndex=3  ColSpan=One column  ColStartMode=Index  ColStartIndex=4  ColEndMode=Index  ColEndIndex=3
  sphere_xyz1 : Subsystem
  T_0HAND_ax/angle : Outport
  T_0HAND_xyz : Outport  Port=2
  wires:
  Rotation Matrix to VRML Rotation3/1 -> Demux16/1
  Demux16/4 -> Mux6/4
  Demux16/3 -> Mux6/2
  Mux6/1 -> Demux15/1
  Demux15/1 -> Mux7/1
  Demux15/2 -> Mux7/2
  Demux15/3 -> Mux7/3
  Demux15/4 -> Mux7/4
  Demux16/1 -> Gain6/1
  Demux16/2 -> Gain7/1
  Gain6/1 -> Mux6/3
  Gain7/1 -> Mux6/1
  Submatrix3/1 -> Rotation Matrix to VRML Rotation3/1
  Submatrix4/1 -> sphere_xyz1/1(xyz_0)
  Mux7/1 -> T_0HAND_ax/angle/1
  sphere_xyz1/1(xyz_vrl) -> T_0HAND_xyz/1
  T_0HAND/1 -> Submatrix3/1, Submatrix4/1

== system Simulatore_COMAU_UBHAND_IV_manipolazione_interna/VISUALIZATION/Subsystem9 ==
  xyz_fingers : Inport
  Demux : Demux  Outputs=5
  Index : Subsystem
  Little : Subsystem
  Middle : Subsystem
  Ring : Subsystem
  Thumb : Subsystem
  xyz_vrl_little : Outport
  xyz_vrl_ring : Outport  Port=2
  xyz_vrl_middle : Outport  Port=3
  xyz_vrl_thumb : Outport  Port=4
  xyz_vrl_index : Outport  Port=5
  wires:
  Thumb/1(xyz_vrl) -> xyz_vrl_thumb/1
  Index/1(xyz_vrl) -> xyz_vrl_index/1
  Middle/1(xyz_vrl) -> xyz_vrl_middle/1
  Ring/1(xyz_vrl) -> xyz_vrl_ring/1
  Little/1(xyz_vrl) -> xyz_vrl_little/1
  Demux/5 -> Little/1(xyz_0)
  Demux/4 -> Ring/1(xyz_0)
  Demux/3 -> Middle/1(xyz_0)
  Demux/1 -> Thumb/1(xyz_0)
  Demux/2 -> Index/1(xyz_0)
  xyz_fingers/1 -> Demux/1

== system Simulatore_COMAU_UBHAND_IV_manipolazione_interna/Work Space Control/  ==
  contact_tips_xyz : Inport
  fingers_reference_mode : Inport  Port=2
  index_position : Inport  Port=3
  Constant : Constant
  Demux : Demux  Outputs=16
  Display : Display
  Multiport Switch : MultiPortSwitch  Inputs=2  zeroidx=off
  Mux{3..4,6..7} (4 alike) : Mux
  Mux5 : Mux  Inputs=5
  into fingers references : Subsystem
  fingers_tips_xyz : Outport
  wires:
  Constant/1 -> [one]
  into fingers references/1(fingers_xyz) -> Demux/1
  Mux5/1 -> Multiport Switch/2
  contact_tips_xyz/1 -> Multiport Switch/3
  fingers_reference_mode/1 -> Multiport Switch/1
  Multiport Switch/1 -> fingers_tips_xyz/1
  Demux/1 -> Mux3/1
  Demux/2 -> Mux3/2
  Demux/3 -> Mux3/3
  Demux/4 -> Mux3/4
  Demux/5 -> Mux4/1
  Demux/6 -> Mux4/2
  Demux/7 -> Mux4/3
  Demux/8 -> Mux4/4
  Demux/9 -> Mux6/1
  Demux/10 -> Mux6/2
  Demux/11 -> Mux6/3
  Demux/12 -> Mux6/4
  Demux/13 -> Mux7/1
  Demux/14 -> Mux7/2
  Demux/15 -> Mux7/3
  Demux/16 -> Mux7/4
  Mux7/1 -> Mux5/5
  Mux6/1 -> Mux5/4
  Mux4/1 -> Mux5/3
  Mux3/1 -> Mux5/1
  index_position/1 -> Mux5/2, Display/1

== system Simulatore_COMAU_UBHAND_IV_manipolazione_interna/Work Space Control/Calcolo RPY mano ==
  q_comau : Inport
  wrist_angles_des : Inport  Port=2
  Add : Sum  Inputs=-+-+
  Add1 : Sum
  Constant : Constant  Value=90
  Demux : Demux  Outputs=6
  Demux1 : Demux  Outputs=2
  roll_mano : Outport
  pitch_mano : Outport  Port=2
  yaw_mano : Outport  Port=3
  wires:
  q_comau/1 -> Demux/1
  wrist_angles_des/1 -> Demux1/1
  Add/1 -> pitch_mano/1
  Demux/2 -> Add/2
  Constant/1 -> Add/1
  Demux/3 -> Add/3
  Demux1/2 -> Add/4
  Demux/1 -> yaw_mano/1
  Add1/1 -> roll_mano/1
  Demux/4 -> Add1/1
  Demux/6 -> Add1/2
